simulink model "cddDcmApp"  (R2022b)
settings: Solver=FixedStepDiscrete  StartTime=0.0  StopTime=10.0  FixedStep=0.02  RelTol=1e-3
undefined symbols (not defined in the file or in its folder): CCPFaultType, DcChlType, HFAChlType, IcuChannelType, MtrRoutStaEnum, P_SignalPartType, P_Std_ReturnType, PowerChlType, SrsAdChlType, VGAChlType
(part 8 of 13)

== system cddDcmApp/DataServices_DID_D928_DID_D928_3_ADC_value_Upper_Endstop_at_100/DataServices_DID_D928_DID_D928_3_ADC_value_Upper_Endstop_at_100_ReadData_sys ==
  function : TriggerPort  TriggerType=function-call  IsSimulinkFunction=on  FunctionName=DataServices_DID_D928_DID_D928_3_ADC_value_Upper_Endstop_at_100_ReadData  VariantControl=(inherit)
  Ground_1 : Ground
  Ground_2 : Ground
  Terminator_1 : Terminator
  OpStatus : ArgIn  OutDataTypeStr=Enum: Dcm_OpStatusType  ArgumentName=OpStatus
  Data : ArgOut  OutDataTypeStr=uint8  ArgumentName=Data
  ERR : ArgOut  Port=2  OutDataTypeStr=Std_ReturnType  ArgumentName=ERR
  wires:
  OpStatus/1 -> Terminator_1/1
  Ground_1/1 -> Data/1
  Ground_2/1 -> ERR/1

== system cddDcmApp/DataServices_DID_D928_DID_D928_3_ADC_value_Upper_Endstop_at_100/DataServices_DID_D928_DID_D928_3_ADC_value_Upper_Endstop_at_100_WriteData_sys ==
  function : TriggerPort  TriggerType=function-call  IsSimulinkFunction=on  FunctionName=DataServices_DID_D928_DID_D928_3_ADC_value_Upper_Endstop_at_100_WriteData  VariantControl=(inherit)
  Ground_1 : Ground
  Ground_2 : Ground
  Terminator_1 : Terminator
  Terminator_2 : Terminator
  Data : ArgIn  OutDataTypeStr=uint8  PortDimensions=2  ArgumentName=Data
  OpStatus : ArgIn  Port=2  OutDataTypeStr=Enum: Dcm_OpStatusType  ArgumentName=OpStatus
  ErrorCode : ArgOut  OutDataTypeStr=Enum: Dcm_NegativeResponseCodeType  ArgumentName=ErrorCode
  ERR : ArgOut  Port=2  OutDataTypeStr=Std_ReturnType  ArgumentName=ERR
  wires:
  Data/1 -> Terminator_1/1
  OpStatus/1 -> Terminator_2/1
  Ground_1/1 -> ErrorCode/1
  Ground_2/1 -> ERR/1

== system cddDcmApp/DataServices_DID_D929_ComplexStructure_2/DataServices_DID_D929_ComplexStructure_2_ReadData_sys ==
  function : TriggerPort  TriggerType=function-call  IsSimulinkFunction=on  FunctionName=DataServices_DID_D929_ComplexStructure_2_ReadData  VariantControl=(inherit)
  Ground_1 : Ground
  Ground_2 : Ground
  Terminator_1 : Terminator
  OpStatus : ArgIn  OutDataTypeStr=Enum: Dcm_OpStatusType  ArgumentName=OpStatus
  Data : ArgOut  OutDataTypeStr=uint8  ArgumentName=Data
  ERR : ArgOut  Port=2  OutDataTypeStr=Std_ReturnType  ArgumentName=ERR
  wires:
  OpStatus/1 -> Terminator_1/1
  Ground_1/1 -> Data/1
  Ground_2/1 -> ERR/1

== system cddDcmApp/DataServices_DID_D929_DID_D929_1_Requested_Position/DataServices_DID_D929_DID_D929_1_Requested_Position_ReadData_sys ==
  function : TriggerPort  TriggerType=function-call  IsSimulinkFunction=on  FunctionName=DataServices_DID_D929_DID_D929_1_Requested_Position_ReadData  VariantControl=(inherit)
  Ground_1 : Ground
  Ground_2 : Ground
  Terminator_1 : Terminator
  OpStatus : ArgIn  OutDataTypeStr=Enum: Dcm_OpStatusType  ArgumentName=OpStatus
  Data : ArgOut  OutDataTypeStr=uint8  ArgumentName=Data
  ERR : ArgOut  Port=2  OutDataTypeStr=Std_ReturnType  ArgumentName=ERR
  wires:
  OpStatus/1 -> Terminator_1/1
  Ground_1/1 -> Data/1
  Ground_2/1 -> ERR/1

== system cddDcmApp/DataServices_DID_D929_DID_D929_2_Actual_Position/DataServices_DID_D929_DID_D929_2_Actual_Position_ReadData_sys ==
  function : TriggerPort  TriggerType=function-call  IsSimulinkFunction=on  FunctionName=DataServices_DID_D929_DID_D929_2_Actual_Position_ReadData  VariantControl=(inherit)
  Ground_1 : Ground
  Ground_2 : Ground
  Terminator_1 : Terminator
  OpStatus : ArgIn  OutDataTypeStr=Enum: Dcm_OpStatusType  ArgumentName=OpStatus
  Data : ArgOut  OutDataTypeStr=uint8  ArgumentName=Data
  ERR : ArgOut  Port=2  OutDataTypeStr=Std_ReturnType  ArgumentName=ERR
  wires:
  OpStatus/1 -> Terminator_1/1
  Ground_1/1 -> Data/1
  Ground_2/1 -> ERR/1

== system cddDcmApp/DataServices_DID_D92A_DID_D92A_1_No_Of_Movements/DataServices_DID_D92A_DID_D92A_1_No_Of_Movements_ReadData_sys ==
  function : TriggerPort  TriggerType=function-call  IsSimulinkFunction=on  FunctionName=DataServices_DID_D92A_DID_D92A_1_No_Of_Movements_ReadData  VariantControl=(inherit)
  Ground_1 : Ground
  Ground_2 : Ground
  Terminator_1 : Terminator
  OpStatus : ArgIn  OutDataTypeStr=Enum: Dcm_OpStatusType  ArgumentName=OpStatus
  Data : ArgOut  OutDataTypeStr=uint8  ArgumentName=Data
  ERR : ArgOut  Port=2  OutDataTypeStr=Std_ReturnType  ArgumentName=ERR
  wires:
  OpStatus/1 -> Terminator_1/1
  Ground_1/1 -> Data/1
  Ground_2/1 -> ERR/1

== system cddDcmApp/DataServices_DID_D92A_DID_D92A_1_No_Of_Movements/DataServices_DID_D92A_DID_D92A_1_No_Of_Movements_WriteData_sys ==
  function : TriggerPort  TriggerType=function-call  IsSimulinkFunction=on  FunctionName=DataServices_DID_D92A_DID_D92A_1_No_Of_Movements_WriteData  VariantControl=(inherit)
  Ground_1 : Ground
  Ground_2 : Ground
  Terminator_1 : Terminator
  Terminator_2 : Terminator
  Data : ArgIn  OutDataTypeStr=uint8  PortDimensions=4  ArgumentName=Data
  OpStatus : ArgIn  Port=2  OutDataTypeStr=Enum: Dcm_OpStatusType  ArgumentName=OpStatus
  ErrorCode : ArgOut  OutDataTypeStr=Enum: Dcm_NegativeResponseCodeType  ArgumentName=ErrorCode
  ERR : ArgOut  Port=2  OutDataTypeStr=Std_ReturnType  ArgumentName=ERR
  wires:
  Data/1 -> Terminator_1/1
  OpStatus/1 -> Terminator_2/1
  Ground_1/1 -> ErrorCode/1
  Ground_2/1 -> ERR/1

== system cddDcmApp/DataServices_DID_D92A_DID_D92A_2_ADC_value_Lower_Endstop_at_0/DataServices_DID_D92A_DID_D92A_2_ADC_value_Lower_Endstop_at_0_ReadData_sys ==
  function : TriggerPort  TriggerType=function-call  IsSimulinkFunction=on  FunctionName=DataServices_DID_D92A_DID_D92A_2_ADC_value_Lower_Endstop_at_0_ReadData  VariantControl=(inherit)
  Ground_1 : Ground
  Ground_2 : Ground
  Terminator_1 : Terminator
  OpStatus : ArgIn  OutDataTypeStr=Enum: Dcm_OpStatusType  ArgumentName=OpStatus
  Data : ArgOut  OutDataTypeStr=uint8  ArgumentName=Data
  ERR : ArgOut  Port=2  OutDataTypeStr=Std_ReturnType  ArgumentName=ERR
  wires:
  OpStatus/1 -> Terminator_1/1
  Ground_1/1 -> Data/1
  Ground_2/1 -> ERR/1

== system cddDcmApp/DataServices_DID_D92A_DID_D92A_2_ADC_value_Lower_Endstop_at_0/DataServices_DID_D92A_DID_D92A_2_ADC_value_Lower_Endstop_at_0_WriteData_sys ==
  function : TriggerPort  TriggerType=function-call  IsSimulinkFunction=on  FunctionName=DataServices_DID_D92A_DID_D92A_2_ADC_value_Lower_Endstop_at_0_WriteData  VariantControl=(inherit)
  Ground_1 : Ground
  Ground_2 : Ground
  Terminator_1 : Terminator
  Terminator_2 : Terminator
  Data : ArgIn  OutDataTypeStr=uint8  PortDimensions=2  ArgumentName=Data
  OpStatus : ArgIn  Port=2  OutDataTypeStr=Enum: Dcm_OpStatusType  ArgumentName=OpStatus
  ErrorCode : ArgOut  OutDataTypeStr=Enum: Dcm_NegativeResponseCodeType  ArgumentName=ErrorCode
  ERR : ArgOut  Port=2  OutDataTypeStr=Std_ReturnType  ArgumentName=ERR
  wires:
  Data/1 -> Terminator_1/1
  OpStatus/1 -> Terminator_2/1
  Ground_1/1 -> ErrorCode/1
  Ground_2/1 -> ERR/1

== system cddDcmApp/DataServices_DID_D92A_DID_D92A_3_ADC_value_Upper_Endstop_at_100/DataServices_DID_D92A_DID_D92A_3_ADC_value_Upper_Endstop_at_100_ReadData_sys ==
  function : TriggerPort  TriggerType=function-call  IsSimulinkFunction=on  FunctionName=DataServices_DID_D92A_DID_D92A_3_ADC_value_Upper_Endstop_at_100_ReadData  VariantControl=(inherit)
  Ground_1 : Ground
  Ground_2 : Ground
  Terminator_1 : Terminator
  OpStatus : ArgIn  OutDataTypeStr=Enum: Dcm_OpStatusType  ArgumentName=OpStatus
  Data : ArgOut  OutDataTypeStr=uint8  ArgumentName=Data
  ERR : ArgOut  Port=2  OutDataTypeStr=Std_ReturnType  ArgumentName=ERR
  wires:
  OpStatus/1 -> Terminator_1/1
  Ground_1/1 -> Data/1
  Ground_2/1 -> ERR/1

== system cddDcmApp/DataServices_DID_D92A_DID_D92A_3_ADC_value_Upper_Endstop_at_100/DataServices_DID_D92A_DID_D92A_3_ADC_value_Upper_Endstop_at_100_WriteData_sys ==
  function : TriggerPort  TriggerType=function-call  IsSimulinkFunction=on  FunctionName=DataServices_DID_D92A_DID_D92A_3_ADC_value_Upper_Endstop_at_100_WriteData  VariantControl=(inherit)
  Ground_1 : Ground
  Ground_2 : Ground
  Terminator_1 : Terminator
  Terminator_2 : Terminator
  Data : ArgIn  OutDataTypeStr=uint8  PortDimensions=2  ArgumentName=Data
  OpStatus : ArgIn  Port=2  OutDataTypeStr=Enum: Dcm_OpStatusType  ArgumentName=OpStatus
  ErrorCode : ArgOut  OutDataTypeStr=Enum: Dcm_NegativeResponseCodeType  ArgumentName=ErrorCode
  ERR : ArgOut  Port=2  OutDataTypeStr=Std_ReturnType  ArgumentName=ERR
  wires:
  Data/1 -> Terminator_1/1
  OpStatus/1 -> Terminator_2/1
  Ground_1/1 -> ErrorCode/1
  Ground_2/1 -> ERR/1

== system cddDcmApp/DataServices_DID_D92B_ComplexStructure_2/DataServices_DID_D92B_ComplexStructure_2_ReadData_sys ==
  function : TriggerPort  TriggerType=function-call  IsSimulinkFunction=on  FunctionName=DataServices_DID_D92B_ComplexStructure_2_ReadData  VariantControl=(inherit)
  Ground_1 : Ground
  Ground_2 : Ground
  Terminator_1 : Terminator
  OpStatus : ArgIn  OutDataTypeStr=Enum: Dcm_OpStatusType  ArgumentName=OpStatus
  Data : ArgOut  OutDataTypeStr=uint8  ArgumentName=Data
  ERR : ArgOut  Port=2  OutDataTypeStr=Std_ReturnType  ArgumentName=ERR
  wires:
  OpStatus/1 -> Terminator_1/1
  Ground_1/1 -> Data/1
  Ground_2/1 -> ERR/1

== system cddDcmApp/DataServices_DID_D92B_DID_D92B_1_Requested_Position/DataServices_DID_D92B_DID_D92B_1_Requested_Position_ReadData_sys ==
  function : TriggerPort  TriggerType=function-call  IsSimulinkFunction=on  FunctionName=DataServices_DID_D92B_DID_D92B_1_Requested_Position_ReadData  VariantControl=(inherit)
  Ground_1 : Ground
  Ground_2 : Ground
  Terminator_1 : Terminator
  OpStatus : ArgIn  OutDataTypeStr=Enum: Dcm_OpStatusType  ArgumentName=OpStatus
  Data : ArgOut  OutDataTypeStr=uint8  ArgumentName=Data
  ERR : ArgOut  Port=2  OutDataTypeStr=Std_ReturnType  ArgumentName=ERR
  wires:
  OpStatus/1 -> Terminator_1/1
  Ground_1/1 -> Data/1
  Ground_2/1 -> ERR/1

== system cddDcmApp/DataServices_DID_D92B_DID_D92B_2_Actual_Position/DataServices_DID_D92B_DID_D92B_2_Actual_Position_ReadData_sys ==
  function : TriggerPort  TriggerType=function-call  IsSimulinkFunction=on  FunctionName=DataServices_DID_D92B_DID_D92B_2_Actual_Position_ReadData  VariantControl=(inherit)
  Ground_1 : Ground
  Ground_2 : Ground
  Terminator_1 : Terminator
  OpStatus : ArgIn  OutDataTypeStr=Enum: Dcm_OpStatusType  ArgumentName=OpStatus
  Data : ArgOut  OutDataTypeStr=uint8  ArgumentName=Data
  ERR : ArgOut  Port=2  OutDataTypeStr=Std_ReturnType  ArgumentName=ERR
  wires:
  OpStatus/1 -> Terminator_1/1
  Ground_1/1 -> Data/1
  Ground_2/1 -> ERR/1

== system cddDcmApp/DataServices_DID_D92C_DID_D92C_1_No_Of_Movements/DataServices_DID_D92C_DID_D92C_1_No_Of_Movements_ReadData_sys ==
  function : TriggerPort  TriggerType=function-call  IsSimulinkFunction=on  FunctionName=DataServices_DID_D92C_DID_D92C_1_No_Of_Movements_ReadData  VariantControl=(inherit)
  Ground_1 : Ground
  Ground_2 : Ground
  Terminator_1 : Terminator
  OpStatus : ArgIn  OutDataTypeStr=Enum: Dcm_OpStatusType  ArgumentName=OpStatus
  Data : ArgOut  OutDataTypeStr=uint8  ArgumentName=Data
  ERR : ArgOut  Port=2  OutDataTypeStr=Std_ReturnType  ArgumentName=ERR
  wires:
  OpStatus/1 -> Terminator_1/1
  Ground_1/1 -> Data/1
  Ground_2/1 -> ERR/1

== system cddDcmApp/DataServices_DID_D92C_DID_D92C_1_No_Of_Movements/DataServices_DID_D92C_DID_D92C_1_No_Of_Movements_WriteData_sys ==
  function : TriggerPort  TriggerType=function-call  IsSimulinkFunction=on  FunctionName=DataServices_DID_D92C_DID_D92C_1_No_Of_Movements_WriteData  VariantControl=(inherit)
  Ground_1 : Ground
  Ground_2 : Ground
  Terminator_1 : Terminator
  Terminator_2 : Terminator
  Data : ArgIn  OutDataTypeStr=uint8  PortDimensions=4  ArgumentName=Data
  OpStatus : ArgIn  Port=2  OutDataTypeStr=Enum: Dcm_OpStatusType  ArgumentName=OpStatus
  ErrorCode : ArgOut  OutDataTypeStr=Enum: Dcm_NegativeResponseCodeType  ArgumentName=ErrorCode
  ERR : ArgOut  Port=2  OutDataTypeStr=Std_ReturnType  ArgumentName=ERR
  wires:
  Data/1 -> Terminator_1/1
  OpStatus/1 -> Terminator_2/1
  Ground_1/1 -> ErrorCode/1
  Ground_2/1 -> ERR/1

== system cddDcmApp/DataServices_DID_D92C_DID_D92C_2_ADC_value_Lower_Endstop_at_0/DataServices_DID_D92C_DID_D92C_2_ADC_value_Lower_Endstop_at_0_ReadData_sys ==
  function : TriggerPort  TriggerType=function-call  IsSimulinkFunction=on  FunctionName=DataServices_DID_D92C_DID_D92C_2_ADC_value_Lower_Endstop_at_0_ReadData  VariantControl=(inherit)
  Ground_1 : Ground
  Ground_2 : Ground
  Terminator_1 : Terminator
  OpStatus : ArgIn  OutDataTypeStr=Enum: Dcm_OpStatusType  ArgumentName=OpStatus
  Data : ArgOut  OutDataTypeStr=uint8  ArgumentName=Data
  ERR : ArgOut  Port=2  OutDataTypeStr=Std_ReturnType  ArgumentName=ERR
  wires:
  OpStatus/1 -> Terminator_1/1
  Ground_1/1 -> Data/1
  Ground_2/1 -> ERR/1

== system cddDcmApp/DataServices_DID_D92C_DID_D92C_2_ADC_value_Lower_Endstop_at_0/DataServices_DID_D92C_DID_D92C_2_ADC_value_Lower_Endstop_at_0_WriteData_sys ==
  function : TriggerPort  TriggerType=function-call  IsSimulinkFunction=on  FunctionName=DataServices_DID_D92C_DID_D92C_2_ADC_value_Lower_Endstop_at_0_WriteData  VariantControl=(inherit)
  Ground_1 : Ground
  Ground_2 : Ground
  Terminator_1 : Terminator
  Terminator_2 : Terminator
  Data : ArgIn  OutDataTypeStr=uint8  PortDimensions=2  ArgumentName=Data
  OpStatus : ArgIn  Port=2  OutDataTypeStr=Enum: Dcm_OpStatusType  ArgumentName=OpStatus
  ErrorCode : ArgOut  OutDataTypeStr=Enum: Dcm_NegativeResponseCodeType  ArgumentName=ErrorCode
  ERR : ArgOut  Port=2  OutDataTypeStr=Std_ReturnType  ArgumentName=ERR
  wires:
  Data/1 -> Terminator_1/1
  OpStatus/1 -> Terminator_2/1
  Ground_1/1 -> ErrorCode/1
  Ground_2/1 -> ERR/1

== system cddDcmApp/DataServices_DID_D92C_DID_D92C_3_ADC_value_Upper_Endstop_at_100/DataServices_DID_D92C_DID_D92C_3_ADC_value_Upper_Endstop_at_100_ReadData_sys ==
  function : TriggerPort  TriggerType=function-call  IsSimulinkFunction=on  FunctionName=DataServices_DID_D92C_DID_D92C_3_ADC_value_Upper_Endstop_at_100_ReadData  VariantControl=(inherit)
  Ground_1 : Ground
  Ground_2 : Ground
  Terminator_1 : Terminator
  OpStatus : ArgIn  OutDataTypeStr=Enum: Dcm_OpStatusType  ArgumentName=OpStatus
  Data : ArgOut  OutDataTypeStr=uint8  ArgumentName=Data
  ERR : ArgOut  Port=2  OutDataTypeStr=Std_ReturnType  ArgumentName=ERR
  wires:
  OpStatus/1 -> Terminator_1/1
  Ground_1/1 -> Data/1
  Ground_2/1 -> ERR/1

== system cddDcmApp/DataServices_DID_D92C_DID_D92C_3_ADC_value_Upper_Endstop_at_100/DataServices_DID_D92C_DID_D92C_3_ADC_value_Upper_Endstop_at_100_WriteData_sys ==
  function : TriggerPort  TriggerType=function-call  IsSimulinkFunction=on  FunctionName=DataServices_DID_D92C_DID_D92C_3_ADC_value_Upper_Endstop_at_100_WriteData  VariantControl=(inherit)
  Ground_1 : Ground
  Ground_2 : Ground
  Terminator_1 : Terminator
  Terminator_2 : Terminator
  Data : ArgIn  OutDataTypeStr=uint8  PortDimensions=2  ArgumentName=Data
  OpStatus : ArgIn  Port=2  OutDataTypeStr=Enum: Dcm_OpStatusType  ArgumentName=OpStatus
  ErrorCode : ArgOut  OutDataTypeStr=Enum: Dcm_NegativeResponseCodeType  ArgumentName=ErrorCode
  ERR : ArgOut  Port=2  OutDataTypeStr=Std_ReturnType  ArgumentName=ERR
  wires:
  Data/1 -> Terminator_1/1
  OpStatus/1 -> Terminator_2/1
  Ground_1/1 -> ErrorCode/1
  Ground_2/1 -> ERR/1

== system cddDcmApp/DataServices_DID_D92D_ComplexStructure_2/DataServices_DID_D92D_ComplexStructure_2_ReadData_sys ==
  function : TriggerPort  TriggerType=function-call  IsSimulinkFunction=on  FunctionName=DataServices_DID_D92D_ComplexStructure_2_ReadData  VariantControl=(inherit)
  Ground_1 : Ground
  Ground_2 : Ground
  Terminator_1 : Terminator
  OpStatus : ArgIn  OutDataTypeStr=Enum: Dcm_OpStatusType  ArgumentName=OpStatus
  Data : ArgOut  OutDataTypeStr=uint8  ArgumentName=Data
  ERR : ArgOut  Port=2  OutDataTypeStr=Std_ReturnType  ArgumentName=ERR
  wires:
  OpStatus/1 -> Terminator_1/1
  Ground_1/1 -> Data/1
  Ground_2/1 -> ERR/1

== system cddDcmApp/DataServices_DID_D92D_DID_D92D_1_Requested_Position/DataServices_DID_D92D_DID_D92D_1_Requested_Position_ReadData_sys ==
  function : TriggerPort  TriggerType=function-call  IsSimulinkFunction=on  FunctionName=DataServices_DID_D92D_DID_D92D_1_Requested_Position_ReadData  VariantControl=(inherit)
  Ground_1 : Ground
  Ground_2 : Ground
  Terminator_1 : Terminator
  OpStatus : ArgIn  OutDataTypeStr=Enum: Dcm_OpStatusType  ArgumentName=OpStatus
  Data : ArgOut  OutDataTypeStr=uint8  ArgumentName=Data
  ERR : ArgOut  Port=2  OutDataTypeStr=Std_ReturnType  ArgumentName=ERR
  wires:
  OpStatus/1 -> Terminator_1/1
  Ground_1/1 -> Data/1
  Ground_2/1 -> ERR/1

== system cddDcmApp/DataServices_DID_D92D_DID_D92D_2_Actual_Position/DataServices_DID_D92D_DID_D92D_2_Actual_Position_ReadData_sys ==
  function : TriggerPort  TriggerType=function-call  IsSimulinkFunction=on  FunctionName=DataServices_DID_D92D_DID_D92D_2_Actual_Position_ReadData  VariantControl=(inherit)
  Ground_1 : Ground
  Ground_2 : Ground
  Terminator_1 : Terminator
  OpStatus : ArgIn  OutDataTypeStr=Enum: Dcm_OpStatusType  ArgumentName=OpStatus
  Data : ArgOut  OutDataTypeStr=uint8  ArgumentName=Data
  ERR : ArgOut  Port=2  OutDataTypeStr=Std_ReturnType  ArgumentName=ERR
  wires:
  OpStatus/1 -> Terminator_1/1
  Ground_1/1 -> Data/1
  Ground_2/1 -> ERR/1

== system cddDcmApp/DataServices_DID_D92E_DID_D92E_1_No_Of_Movements/DataServices_DID_D92E_DID_D92E_1_No_Of_Movements_ReadData_sys ==
  function : TriggerPort  TriggerType=function-call  IsSimulinkFunction=on  FunctionName=DataServices_DID_D92E_DID_D92E_1_No_Of_Movements_ReadData  VariantControl=(inherit)
  Ground_1 : Ground
  Ground_2 : Ground
  Terminator_1 : Terminator
  OpStatus : ArgIn  OutDataTypeStr=Enum: Dcm_OpStatusType  ArgumentName=OpStatus
  Data : ArgOut  OutDataTypeStr=uint8  ArgumentName=Data
  ERR : ArgOut  Port=2  OutDataTypeStr=Std_ReturnType  ArgumentName=ERR
  wires:
  OpStatus/1 -> Terminator_1/1
  Ground_1/1 -> Data/1
  Ground_2/1 -> ERR/1

== system cddDcmApp/DataServices_DID_D92E_DID_D92E_1_No_Of_Movements/DataServices_DID_D92E_DID_D92E_1_No_Of_Movements_WriteData_sys ==
  function : TriggerPort  TriggerType=function-call  IsSimulinkFunction=on  FunctionName=DataServices_DID_D92E_DID_D92E_1_No_Of_Movements_WriteData  VariantControl=(inherit)
  Ground_1 : Ground
  Ground_2 : Ground
  Terminator_1 : Terminator
  Terminator_2 : Terminator
  Data : ArgIn  OutDataTypeStr=uint8  PortDimensions=4  ArgumentName=Data
  OpStatus : ArgIn  Port=2  OutDataTypeStr=Enum: Dcm_OpStatusType  ArgumentName=OpStatus
  ErrorCode : ArgOut  OutDataTypeStr=Enum: Dcm_NegativeResponseCodeType  ArgumentName=ErrorCode
  ERR : ArgOut  Port=2  OutDataTypeStr=Std_ReturnType  ArgumentName=ERR
  wires:
  Data/1 -> Terminator_1/1
  OpStatus/1 -> Terminator_2/1
  Ground_1/1 -> ErrorCode/1
  Ground_2/1 -> ERR/1

== system cddDcmApp/DataServices_DID_D92E_DID_D92E_2_ADC_value_Lower_Endstop_at_0/DataServices_DID_D92E_DID_D92E_2_ADC_value_Lower_Endstop_at_0_ReadData_sys ==
  function : TriggerPort  TriggerType=function-call  IsSimulinkFunction=on  FunctionName=DataServices_DID_D92E_DID_D92E_2_ADC_value_Lower_Endstop_at_0_ReadData  VariantControl=(inherit)
  Ground_1 : Ground
  Ground_2 : Ground
  Terminator_1 : Terminator
  OpStatus : ArgIn  OutDataTypeStr=Enum: Dcm_OpStatusType  ArgumentName=OpStatus
  Data : ArgOut  OutDataTypeStr=uint8  ArgumentName=Data
  ERR : ArgOut  Port=2  OutDataTypeStr=Std_ReturnType  ArgumentName=ERR
  wires:
  OpStatus/1 -> Terminator_1/1
  Ground_1/1 -> Data/1
  Ground_2/1 -> ERR/1

== system cddDcmApp/DataServices_DID_D92E_DID_D92E_2_ADC_value_Lower_Endstop_at_0/DataServices_DID_D92E_DID_D92E_2_ADC_value_Lower_Endstop_at_0_WriteData_sys ==
  function : TriggerPort  TriggerType=function-call  IsSimulinkFunction=on  FunctionName=DataServices_DID_D92E_DID_D92E_2_ADC_value_Lower_Endstop_at_0_WriteData  VariantControl=(inherit)
  Ground_1 : Ground
  Ground_2 : Ground
  Terminator_1 : Terminator
  Terminator_2 : Terminator
  Data : ArgIn  OutDataTypeStr=uint8  PortDimensions=2  ArgumentName=Data
  OpStatus : ArgIn  Port=2  OutDataTypeStr=Enum: Dcm_OpStatusType  ArgumentName=OpStatus
  ErrorCode : ArgOut  OutDataTypeStr=Enum: Dcm_NegativeResponseCodeType  ArgumentName=ErrorCode
  ERR : ArgOut  Port=2  OutDataTypeStr=Std_ReturnType  ArgumentName=ERR
  wires:
  Data/1 -> Terminator_1/1
  OpStatus/1 -> Terminator_2/1
  Ground_1/1 -> ErrorCode/1
  Ground_2/1 -> ERR/1

== system cddDcmApp/DataServices_DID_D92E_DID_D92E_3_ADC_value_Upper_Endstop_at_100/DataServices_DID_D92E_DID_D92E_3_ADC_value_Upper_Endstop_at_100_ReadData_sys ==
  function : TriggerPort  TriggerType=function-call  IsSimulinkFunction=on  FunctionName=DataServices_DID_D92E_DID_D92E_3_ADC_value_Upper_Endstop_at_100_ReadData  VariantControl=(inherit)
  Ground_1 : Ground
  Ground_2 : Ground
  Terminator_1 : Terminator
  OpStatus : ArgIn  OutDataTypeStr=Enum: Dcm_OpStatusType  ArgumentName=OpStatus
  Data : ArgOut  OutDataTypeStr=uint8  ArgumentName=Data
  ERR : ArgOut  Port=2  OutDataTypeStr=Std_ReturnType  ArgumentName=ERR
  wires:
  OpStatus/1 -> Terminator_1/1
  Ground_1/1 -> Data/1
  Ground_2/1 -> ERR/1

== system cddDcmApp/DataServices_DID_D92E_DID_D92E_3_ADC_value_Upper_Endstop_at_100/DataServices_DID_D92E_DID_D92E_3_ADC_value_Upper_Endstop_at_100_WriteData_sys ==
  function : TriggerPort  TriggerType=function-call  IsSimulinkFunction=on  FunctionName=DataServices_DID_D92E_DID_D92E_3_ADC_value_Upper_Endstop_at_100_WriteData  VariantControl=(inherit)
  Ground_1 : Ground
  Ground_2 : Ground
  Terminator_1 : Terminator
  Terminator_2 : Terminator
  Data : ArgIn  OutDataTypeStr=uint8  PortDimensions=2  ArgumentName=Data
  OpStatus : ArgIn  Port=2  OutDataTypeStr=Enum: Dcm_OpStatusType  ArgumentName=OpStatus
  ErrorCode : ArgOut  OutDataTypeStr=Enum: Dcm_NegativeResponseCodeType  ArgumentName=ErrorCode
  ERR : ArgOut  Port=2  OutDataTypeStr=Std_ReturnType  ArgumentName=ERR
  wires:
  Data/1 -> Terminator_1/1
  OpStatus/1 -> Terminator_2/1
  Ground_1/1 -> ErrorCode/1
  Ground_2/1 -> ERR/1

== system cddDcmApp/DataServices_DID_D92F_ComplexStructure_2/DataServices_DID_D92F_ComplexStructure_2_ReadData_sys ==
  function : TriggerPort  TriggerType=function-call  IsSimulinkFunction=on  FunctionName=DataServices_DID_D92F_ComplexStructure_2_ReadData  VariantControl=(inherit)
  Ground_1 : Ground
  Ground_2 : Ground
  Terminator_1 : Terminator
  OpStatus : ArgIn  OutDataTypeStr=Enum: Dcm_OpStatusType  ArgumentName=OpStatus
  Data : ArgOut  OutDataTypeStr=uint8  ArgumentName=Data
  ERR : ArgOut  Port=2  OutDataTypeStr=Std_ReturnType  ArgumentName=ERR
  wires:
  OpStatus/1 -> Terminator_1/1
  Ground_1/1 -> Data/1
  Ground_2/1 -> ERR/1

== system cddDcmApp/DataServices_DID_D92F_DID_D92F_1_Requested_Position/DataServices_DID_D92F_DID_D92F_1_Requested_Position_ReadData_sys ==
  function : TriggerPort  TriggerType=function-call  IsSimulinkFunction=on  FunctionName=DataServices_DID_D92F_DID_D92F_1_Requested_Position_ReadData  VariantControl=(inherit)
  Ground_1 : Ground
  Ground_2 : Ground
  Terminator_1 : Terminator
  OpStatus : ArgIn  OutDataTypeStr=Enum: Dcm_OpStatusType  ArgumentName=OpStatus
  Data : ArgOut  OutDataTypeStr=uint8  ArgumentName=Data
  ERR : ArgOut  Port=2  OutDataTypeStr=Std_ReturnType  ArgumentName=ERR
  wires:
  OpStatus/1 -> Terminator_1/1
  Ground_1/1 -> Data/1
  Ground_2/1 -> ERR/1

== system cddDcmApp/DataServices_DID_D92F_DID_D92F_2_Actual_Position/DataServices_DID_D92F_DID_D92F_2_Actual_Position_ReadData_sys ==
  function : TriggerPort  TriggerType=function-call  IsSimulinkFunction=on  FunctionName=DataServices_DID_D92F_DID_D92F_2_Actual_Position_ReadData  VariantControl=(inherit)
  Ground_1 : Ground
  Ground_2 : Ground
  Terminator_1 : Terminator
  OpStatus : ArgIn  OutDataTypeStr=Enum: Dcm_OpStatusType  ArgumentName=OpStatus
  Data : ArgOut  OutDataTypeStr=uint8  ArgumentName=Data
  ERR : ArgOut  Port=2  OutDataTypeStr=Std_ReturnType  ArgumentName=ERR
  wires:
  OpStatus/1 -> Terminator_1/1
  Ground_1/1 -> Data/1
  Ground_2/1 -> ERR/1

== system cddDcmApp/DataServices_DID_D930_DID_D930_1_No_Of_Movements/DataServices_DID_D930_DID_D930_1_No_Of_Movements_ReadData_sys ==
  function : TriggerPort  TriggerType=function-call  IsSimulinkFunction=on  FunctionName=DataServices_DID_D930_DID_D930_1_No_Of_Movements_ReadData  VariantControl=(inherit)
  Ground_1 : Ground
  Ground_2 : Ground
  Terminator_1 : Terminator
  OpStatus : ArgIn  OutDataTypeStr=Enum: Dcm_OpStatusType  ArgumentName=OpStatus
  Data : ArgOut  OutDataTypeStr=uint8  ArgumentName=Data
  ERR : ArgOut  Port=2  OutDataTypeStr=Std_ReturnType  ArgumentName=ERR
  wires:
  OpStatus/1 -> Terminator_1/1
  Ground_1/1 -> Data/1
  Ground_2/1 -> ERR/1

== system cddDcmApp/DataServices_DID_D930_DID_D930_1_No_Of_Movements/DataServices_DID_D930_DID_D930_1_No_Of_Movements_WriteData_sys ==
  function : TriggerPort  TriggerType=function-call  IsSimulinkFunction=on  FunctionName=DataServices_DID_D930_DID_D930_1_No_Of_Movements_WriteData  VariantControl=(inherit)
  Ground_1 : Ground
  Ground_2 : Ground
  Terminator_1 : Terminator
  Terminator_2 : Terminator
  Data : ArgIn  OutDataTypeStr=uint8  PortDimensions=4  ArgumentName=Data
  OpStatus : ArgIn  Port=2  OutDataTypeStr=Enum: Dcm_OpStatusType  ArgumentName=OpStatus
  ErrorCode : ArgOut  OutDataTypeStr=Enum: Dcm_NegativeResponseCodeType  ArgumentName=ErrorCode
  ERR : ArgOut  Port=2  OutDataTypeStr=Std_ReturnType  ArgumentName=ERR
  wires:
  Data/1 -> Terminator_1/1
  OpStatus/1 -> Terminator_2/1
  Ground_1/1 -> ErrorCode/1
  Ground_2/1 -> ERR/1

== system cddDcmApp/DataServices_DID_D930_DID_D930_2_ADC_value_Lower_Endstop_at_0/DataServices_DID_D930_DID_D930_2_ADC_value_Lower_Endstop_at_0_ReadData_sys ==
  function : TriggerPort  TriggerType=function-call  IsSimulinkFunction=on  FunctionName=DataServices_DID_D930_DID_D930_2_ADC_value_Lower_Endstop_at_0_ReadData  VariantControl=(inherit)
  Ground_1 : Ground
  Ground_2 : Ground
  Terminator_1 : Terminator
  OpStatus : ArgIn  OutDataTypeStr=Enum: Dcm_OpStatusType  ArgumentName=OpStatus
  Data : ArgOut  OutDataTypeStr=uint8  ArgumentName=Data
  ERR : ArgOut  Port=2  OutDataTypeStr=Std_ReturnType  ArgumentName=ERR
  wires:
  OpStatus/1 -> Terminator_1/1
  Ground_1/1 -> Data/1
  Ground_2/1 -> ERR/1

== system cddDcmApp/DataServices_DID_D930_DID_D930_2_ADC_value_Lower_Endstop_at_0/DataServices_DID_D930_DID_D930_2_ADC_value_Lower_Endstop_at_0_WriteData_sys ==
  function : TriggerPort  TriggerType=function-call  IsSimulinkFunction=on  FunctionName=DataServices_DID_D930_DID_D930_2_ADC_value_Lower_Endstop_at_0_WriteData  VariantControl=(inherit)
  Ground_1 : Ground
  Ground_2 : Ground
  Terminator_1 : Terminator
  Terminator_2 : Terminator
  Data : ArgIn  OutDataTypeStr=uint8  PortDimensions=2  ArgumentName=Data
  OpStatus : ArgIn  Port=2  OutDataTypeStr=Enum: Dcm_OpStatusType  ArgumentName=OpStatus
  ErrorCode : ArgOut  OutDataTypeStr=Enum: Dcm_NegativeResponseCodeType  ArgumentName=ErrorCode
  ERR : ArgOut  Port=2  OutDataTypeStr=Std_ReturnType  ArgumentName=ERR
  wires:
  Data/1 -> Terminator_1/1
  OpStatus/1 -> Terminator_2/1
  Ground_1/1 -> ErrorCode/1
  Ground_2/1 -> ERR/1

== system cddDcmApp/DataServices_DID_D930_DID_D930_3_ADC_value_Upper_Endstop_at_100/DataServices_DID_D930_DID_D930_3_ADC_value_Upper_Endstop_at_100_ReadData_sys ==
  function : TriggerPort  TriggerType=function-call  IsSimulinkFunction=on  FunctionName=DataServices_DID_D930_DID_D930_3_ADC_value_Upper_Endstop_at_100_ReadData  VariantControl=(inherit)
  Ground_1 : Ground
  Ground_2 : Ground
  Terminator_1 : Terminator
  OpStatus : ArgIn  OutDataTypeStr=Enum: Dcm_OpStatusType  ArgumentName=OpStatus
  Data : ArgOut  OutDataTypeStr=uint8  ArgumentName=Data
  ERR : ArgOut  Port=2  OutDataTypeStr=Std_ReturnType  ArgumentName=ERR
  wires:
  OpStatus/1 -> Terminator_1/1
  Ground_1/1 -> Data/1
  Ground_2/1 -> ERR/1

== system cddDcmApp/DataServices_DID_D930_DID_D930_3_ADC_value_Upper_Endstop_at_100/DataServices_DID_D930_DID_D930_3_ADC_value_Upper_Endstop_at_100_WriteData_sys ==
  function : TriggerPort  TriggerType=function-call  IsSimulinkFunction=on  FunctionName=DataServices_DID_D930_DID_D930_3_ADC_value_Upper_Endstop_at_100_WriteData  VariantControl=(inherit)
  Ground_1 : Ground
  Ground_2 : Ground
  Terminator_1 : Terminator
  Terminator_2 : Terminator
  Data : ArgIn  OutDataTypeStr=uint8  PortDimensions=2  ArgumentName=Data
  OpStatus : ArgIn  Port=2  OutDataTypeStr=Enum: Dcm_OpStatusType  ArgumentName=OpStatus
  ErrorCode : ArgOut  OutDataTypeStr=Enum: Dcm_NegativeResponseCodeType  ArgumentName=ErrorCode
  ERR : ArgOut  Port=2  OutDataTypeStr=Std_ReturnType  ArgumentName=ERR
  wires:
  Data/1 -> Terminator_1/1
  OpStatus/1 -> Terminator_2/1
  Ground_1/1 -> ErrorCode/1
  Ground_2/1 -> ERR/1

== system cddDcmApp/DataServices_DID_D931_ComplexStructure_2/DataServices_DID_D931_ComplexStructure_2_ReadData_sys ==
  function : TriggerPort  TriggerType=function-call  IsSimulinkFunction=on  FunctionName=DataServices_DID_D931_ComplexStructure_2_ReadData  VariantControl=(inherit)
  Ground_1 : Ground
  Ground_2 : Ground
  Terminator_1 : Terminator
  OpStatus : ArgIn  OutDataTypeStr=Enum: Dcm_OpStatusType  ArgumentName=OpStatus
  Data : ArgOut  OutDataTypeStr=uint8  ArgumentName=Data
  ERR : ArgOut  Port=2  OutDataTypeStr=Std_ReturnType  ArgumentName=ERR
  wires:
  OpStatus/1 -> Terminator_1/1
  Ground_1/1 -> Data/1
  Ground_2/1 -> ERR/1

== system cddDcmApp/DataServices_DID_D931_DID_D931_1_Requested_Position/DataServices_DID_D931_DID_D931_1_Requested_Position_ReadData_sys ==
  function : TriggerPort  TriggerType=function-call  IsSimulinkFunction=on  FunctionName=DataServices_DID_D931_DID_D931_1_Requested_Position_ReadData  VariantControl=(inherit)
  Ground_1 : Ground
  Ground_2 : Ground
  Terminator_1 : Terminator
  OpStatus : ArgIn  OutDataTypeStr=Enum: Dcm_OpStatusType  ArgumentName=OpStatus
  Data : ArgOut  OutDataTypeStr=uint8  ArgumentName=Data
  ERR : ArgOut  Port=2  OutDataTypeStr=Std_ReturnType  ArgumentName=ERR
  wires:
  OpStatus/1 -> Terminator_1/1
  Ground_1/1 -> Data/1
  Ground_2/1 -> ERR/1

== system cddDcmApp/DataServices_DID_D931_DID_D931_2_Actual_Position/DataServices_DID_D931_DID_D931_2_Actual_Position_ReadData_sys ==
  function : TriggerPort  TriggerType=function-call  IsSimulinkFunction=on  FunctionName=DataServices_DID_D931_DID_D931_2_Actual_Position_ReadData  VariantControl=(inherit)
  Ground_1 : Ground
  Ground_2 : Ground
  Terminator_1 : Terminator
  OpStatus : ArgIn  OutDataTypeStr=Enum: Dcm_OpStatusType  ArgumentName=OpStatus
  Data : ArgOut  OutDataTypeStr=uint8  ArgumentName=Data
  ERR : ArgOut  Port=2  OutDataTypeStr=Std_ReturnType  ArgumentName=ERR
  wires:
  OpStatus/1 -> Terminator_1/1
  Ground_1/1 -> Data/1
  Ground_2/1 -> ERR/1

== system cddDcmApp/DataServices_DID_D933_DID_D933_1_step_Range/DataServices_DID_D933_DID_D933_1_step_Range_ReadData_sys ==
  function : TriggerPort  TriggerType=function-call  IsSimulinkFunction=on  FunctionName=DataServices_DID_D933_DID_D933_1_step_Range_ReadData  VariantControl=(inherit)
  Ground_1 : Ground
  Ground_2 : Ground
  Terminator_1 : Terminator
  OpStatus : ArgIn  OutDataTypeStr=Enum: Dcm_OpStatusType  ArgumentName=OpStatus
  Data : ArgOut  OutDataTypeStr=uint8  ArgumentName=Data
  ERR : ArgOut  Port=2  OutDataTypeStr=Std_ReturnType  ArgumentName=ERR
  wires:
  OpStatus/1 -> Terminator_1/1
  Ground_1/1 -> Data/1
  Ground_2/1 -> ERR/1

== system cddDcmApp/DataServices_DID_D933_DID_D933_1_step_Range/DataServices_DID_D933_DID_D933_1_step_Range_WriteData_sys ==
  function : TriggerPort  TriggerType=function-call  IsSimulinkFunction=on  FunctionName=DataServices_DID_D933_DID_D933_1_step_Range_WriteData  VariantControl=(inherit)
  Ground_1 : Ground
  Ground_2 : Ground
  Terminator_1 : Terminator
  Terminator_2 : Terminator
  Data : ArgIn  OutDataTypeStr=uint8  PortDimensions=2  ArgumentName=Data
  OpStatus : ArgIn  Port=2  OutDataTypeStr=Enum: Dcm_OpStatusType  ArgumentName=OpStatus
  ErrorCode : ArgOut  OutDataTypeStr=Enum: Dcm_NegativeResponseCodeType  ArgumentName=ErrorCode
  ERR : ArgOut  Port=2  OutDataTypeStr=Std_ReturnType  ArgumentName=ERR
  wires:
  Data/1 -> Terminator_1/1
  OpStatus/1 -> Terminator_2/1
  Ground_1/1 -> ErrorCode/1
  Ground_2/1 -> ERR/1

== system cddDcmApp/DataServices_DID_D933_DID_D933_2_No_Of_Movements/DataServices_DID_D933_DID_D933_2_No_Of_Movements_ReadData_sys ==
  function : TriggerPort  TriggerType=function-call  IsSimulinkFunction=on  FunctionName=DataServices_DID_D933_DID_D933_2_No_Of_Movements_ReadData  VariantControl=(inherit)
  Ground_1 : Ground
  Ground_2 : Ground
  Terminator_1 : Terminator
  OpStatus : ArgIn  OutDataTypeStr=Enum: Dcm_OpStatusType  ArgumentName=OpStatus
  Data : ArgOut  OutDataTypeStr=uint8  ArgumentName=Data
  ERR : ArgOut  Port=2  OutDataTypeStr=Std_ReturnType  ArgumentName=ERR
  wires:
  OpStatus/1 -> Terminator_1/1
  Ground_1/1 -> Data/1
  Ground_2/1 -> ERR/1

== system cddDcmApp/DataServices_DID_D933_DID_D933_2_No_Of_Movements/DataServices_DID_D933_DID_D933_2_No_Of_Movements_WriteData_sys ==
  function : TriggerPort  TriggerType=function-call  IsSimulinkFunction=on  FunctionName=DataServices_DID_D933_DID_D933_2_No_Of_Movements_WriteData  VariantControl=(inherit)
  Ground_1 : Ground
  Ground_2 : Ground
  Terminator_1 : Terminator
  Terminator_2 : Terminator
  Data : ArgIn  OutDataTypeStr=uint8  PortDimensions=4  ArgumentName=Data
  OpStatus : ArgIn  Port=2  OutDataTypeStr=Enum: Dcm_OpStatusType  ArgumentName=OpStatus
  ErrorCode : ArgOut  OutDataTypeStr=Enum: Dcm_NegativeResponseCodeType  ArgumentName=ErrorCode
  ERR : ArgOut  Port=2  OutDataTypeStr=Std_ReturnType  ArgumentName=ERR
  wires:
  Data/1 -> Terminator_1/1
  OpStatus/1 -> Terminator_2/1
  Ground_1/1 -> ErrorCode/1
  Ground_2/1 -> ERR/1

== system cddDcmApp/DataServices_DID_D934_ComplexStructure_2/DataServices_DID_D934_ComplexStructure_2_ReadData_sys ==
  function : TriggerPort  TriggerType=function-call  IsSimulinkFunction=on  FunctionName=DataServices_DID_D934_ComplexStructure_2_ReadData  VariantControl=(inherit)
  Ground_1 : Ground
  Ground_2 : Ground
  Data : ArgOut  OutDataTypeStr=uint8  ArgumentName=Data
  ERR : ArgOut  Port=2  OutDataTypeStr=Std_ReturnType  ArgumentName=ERR
  wires:
  Ground_1/1 -> Data/1
  Ground_2/1 -> ERR/1

== system cddDcmApp/DataServices_DID_D934_DID_D934_1_Requested_Position/DataServices_DID_D934_DID_D934_1_Requested_Position_ReadData_sys ==
  function : TriggerPort  TriggerType=function-call  IsSimulinkFunction=on  FunctionName=DataServices_DID_D934_DID_D934_1_Requested_Position_ReadData  VariantControl=(inherit)
  Ground_1 : Ground
  Ground_2 : Ground
  Data : ArgOut  OutDataTypeStr=uint8  ArgumentName=Data
  ERR : ArgOut  Port=2  OutDataTypeStr=Std_ReturnType  ArgumentName=ERR
  wires:
  Ground_1/1 -> Data/1
  Ground_2/1 -> ERR/1

== system cddDcmApp/DataServices_DID_D934_DID_D934_2_Actual_Position/DataServices_DID_D934_DID_D934_2_Actual_Position_ReadData_sys ==
  function : TriggerPort  TriggerType=function-call  IsSimulinkFunction=on  FunctionName=DataServices_DID_D934_DID_D934_2_Actual_Position_ReadData  VariantControl=(inherit)
  Ground_1 : Ground
  Ground_2 : Ground
  Data : ArgOut  OutDataTypeStr=uint8  ArgumentName=Data
  ERR : ArgOut  Port=2  OutDataTypeStr=Std_ReturnType  ArgumentName=ERR
  wires:
  Ground_1/1 -> Data/1
  Ground_2/1 -> ERR/1

== system cddDcmApp/DataServices_DID_D935_DID_D935_1_step_Range/DataServices_DID_D935_DID_D935_1_step_Range_ReadData_sys ==
  function : TriggerPort  TriggerType=function-call  IsSimulinkFunction=on  FunctionName=DataServices_DID_D935_DID_D935_1_step_Range_ReadData  VariantControl=(inherit)
  Ground_1 : Ground
  Ground_2 : Ground
  Terminator_1 : Terminator
  OpStatus : ArgIn  OutDataTypeStr=Enum: Dcm_OpStatusType  ArgumentName=OpStatus
  Data : ArgOut  OutDataTypeStr=uint8  ArgumentName=Data
  ERR : ArgOut  Port=2  OutDataTypeStr=Std_ReturnType  ArgumentName=ERR
  wires:
  OpStatus/1 -> Terminator_1/1
  Ground_1/1 -> Data/1
  Ground_2/1 -> ERR/1

== system cddDcmApp/DataServices_DID_D935_DID_D935_1_step_Range/DataServices_DID_D935_DID_D935_1_step_Range_WriteData_sys ==
  function : TriggerPort  TriggerType=function-call  IsSimulinkFunction=on  FunctionName=DataServices_DID_D935_DID_D935_1_step_Range_WriteData  VariantControl=(inherit)
  Ground_1 : Ground
  Ground_2 : Ground
  Terminator_1 : Terminator
  Terminator_2 : Terminator
  Data : ArgIn  OutDataTypeStr=uint8  PortDimensions=2  ArgumentName=Data
  OpStatus : ArgIn  Port=2  OutDataTypeStr=Enum: Dcm_OpStatusType  ArgumentName=OpStatus
  ErrorCode : ArgOut  OutDataTypeStr=Enum: Dcm_NegativeResponseCodeType  ArgumentName=ErrorCode
  ERR : ArgOut  Port=2  OutDataTypeStr=Std_ReturnType  ArgumentName=ERR
  wires:
  Data/1 -> Terminator_1/1
  OpStatus/1 -> Terminator_2/1
  Ground_1/1 -> ErrorCode/1
  Ground_2/1 -> ERR/1

== system cddDcmApp/DataServices_DID_D935_DID_D935_2_No_Of_Movements/DataServices_DID_D935_DID_D935_2_No_Of_Movements_ReadData_sys ==
  function : TriggerPort  TriggerType=function-call  IsSimulinkFunction=on  FunctionName=DataServices_DID_D935_DID_D935_2_No_Of_Movements_ReadData  VariantControl=(inherit)
  Ground_1 : Ground
  Ground_2 : Ground
  Terminator_1 : Terminator
  OpStatus : ArgIn  OutDataTypeStr=Enum: Dcm_OpStatusType  ArgumentName=OpStatus
  Data : ArgOut  OutDataTypeStr=uint8  ArgumentName=Data
  ERR : ArgOut  Port=2  OutDataTypeStr=Std_ReturnType  ArgumentName=ERR
  wires:
  OpStatus/1 -> Terminator_1/1
  Ground_1/1 -> Data/1
  Ground_2/1 -> ERR/1

== system cddDcmApp/DataServices_DID_D935_DID_D935_2_No_Of_Movements/DataServices_DID_D935_DID_D935_2_No_Of_Movements_WriteData_sys ==
  function : TriggerPort  TriggerType=function-call  IsSimulinkFunction=on  FunctionName=DataServices_DID_D935_DID_D935_2_No_Of_Movements_WriteData  VariantControl=(inherit)
  Ground_1 : Ground
  Ground_2 : Ground
  Terminator_1 : Terminator
  Terminator_2 : Terminator
  Data : ArgIn  OutDataTypeStr=uint8  PortDimensions=4  ArgumentName=Data
  OpStatus : ArgIn  Port=2  OutDataTypeStr=Enum: Dcm_OpStatusType  ArgumentName=OpStatus
  ErrorCode : ArgOut  OutDataTypeStr=Enum: Dcm_NegativeResponseCodeType  ArgumentName=ErrorCode
  ERR : ArgOut  Port=2  OutDataTypeStr=Std_ReturnType  ArgumentName=ERR
  wires:
  Data/1 -> Terminator_1/1
  OpStatus/1 -> Terminator_2/1
  Ground_1/1 -> ErrorCode/1
  Ground_2/1 -> ERR/1

== system cddDcmApp/DataServices_DID_D936_ComplexStructure_2/DataServices_DID_D936_ComplexStructure_2_ReadData_sys ==
  function : TriggerPort  TriggerType=function-call  IsSimulinkFunction=on  FunctionName=DataServices_DID_D936_ComplexStructure_2_ReadData  VariantControl=(inherit)
  Ground_1 : Ground
  Ground_2 : Ground
  Terminator_1 : Terminator
  OpStatus : ArgIn  OutDataTypeStr=Enum: Dcm_OpStatusType  ArgumentName=OpStatus
  Data : ArgOut  OutDataTypeStr=uint8  ArgumentName=Data
  ERR : ArgOut  Port=2  OutDataTypeStr=Std_ReturnType  ArgumentName=ERR
  wires:
  OpStatus/1 -> Terminator_1/1
  Ground_1/1 -> Data/1
  Ground_2/1 -> ERR/1

== system cddDcmApp/DataServices_DID_D936_DID_D936_1_Requested_Position/DataServices_DID_D936_DID_D936_1_Requested_Position_ReadData_sys ==
  function : TriggerPort  TriggerType=function-call  IsSimulinkFunction=on  FunctionName=DataServices_DID_D936_DID_D936_1_Requested_Position_ReadData  VariantControl=(inherit)
  Ground_1 : Ground
  Ground_2 : Ground
  Terminator_1 : Terminator
  OpStatus : ArgIn  OutDataTypeStr=Enum: Dcm_OpStatusType  ArgumentName=OpStatus
  Data : ArgOut  OutDataTypeStr=uint8  ArgumentName=Data
  ERR : ArgOut  Port=2  OutDataTypeStr=Std_ReturnType  ArgumentName=ERR
  wires:
  OpStatus/1 -> Terminator_1/1
  Ground_1/1 -> Data/1
  Ground_2/1 -> ERR/1

== system cddDcmApp/DataServices_DID_D936_DID_D936_2_Actual_Position/DataServices_DID_D936_DID_D936_2_Actual_Position_ReadData_sys ==
  function : TriggerPort  TriggerType=function-call  IsSimulinkFunction=on  FunctionName=DataServices_DID_D936_DID_D936_2_Actual_Position_ReadData  VariantControl=(inherit)
  Ground_1 : Ground
  Ground_2 : Ground
  Terminator_1 : Terminator
  OpStatus : ArgIn  OutDataTypeStr=Enum: Dcm_OpStatusType  ArgumentName=OpStatus
  Data : ArgOut  OutDataTypeStr=uint8  ArgumentName=Data
  ERR : ArgOut  Port=2  OutDataTypeStr=Std_ReturnType  ArgumentName=ERR
  wires:
  OpStatus/1 -> Terminator_1/1
  Ground_1/1 -> Data/1
  Ground_2/1 -> ERR/1

== system cddDcmApp/DataServices_DID_D937_DID_D937_1_step_Range/DataServices_DID_D937_DID_D937_1_step_Range_ReadData_sys ==
  function : TriggerPort  TriggerType=function-call  IsSimulinkFunction=on  FunctionName=DataServices_DID_D937_DID_D937_1_step_Range_ReadData  VariantControl=(inherit)
  Ground_1 : Ground
  Ground_2 : Ground
  Terminator_1 : Terminator
  OpStatus : ArgIn  OutDataTypeStr=Enum: Dcm_OpStatusType  ArgumentName=OpStatus
  Data : ArgOut  OutDataTypeStr=uint8  ArgumentName=Data
  ERR : ArgOut  Port=2  OutDataTypeStr=Std_ReturnType  ArgumentName=ERR
  wires:
  OpStatus/1 -> Terminator_1/1
  Ground_1/1 -> Data/1
  Ground_2/1 -> ERR/1

== system cddDcmApp/DataServices_DID_D937_DID_D937_1_step_Range/DataServices_DID_D937_DID_D937_1_step_Range_WriteData_sys ==
  function : TriggerPort  TriggerType=function-call  IsSimulinkFunction=on  FunctionName=DataServices_DID_D937_DID_D937_1_step_Range_WriteData  VariantControl=(inherit)
  Ground_1 : Ground
  Ground_2 : Ground
  Terminator_1 : Terminator
  Terminator_2 : Terminator
  Data : ArgIn  OutDataTypeStr=uint8  PortDimensions=2  ArgumentName=Data
  OpStatus : ArgIn  Port=2  OutDataTypeStr=Enum: Dcm_OpStatusType  ArgumentName=OpStatus
  ErrorCode : ArgOut  OutDataTypeStr=Enum: Dcm_NegativeResponseCodeType  ArgumentName=ErrorCode
  ERR : ArgOut  Port=2  OutDataTypeStr=Std_ReturnType  ArgumentName=ERR
  wires:
  Data/1 -> Terminator_1/1
  OpStatus/1 -> Terminator_2/1
  Ground_1/1 -> ErrorCode/1
  Ground_2/1 -> ERR/1

== system cddDcmApp/DataServices_DID_D937_DID_D937_2_No_Of_Movements/DataServices_DID_D937_DID_D937_2_No_Of_Movements_ReadData_sys ==
  function : TriggerPort  TriggerType=function-call  IsSimulinkFunction=on  FunctionName=DataServices_DID_D937_DID_D937_2_No_Of_Movements_ReadData  VariantControl=(inherit)
  Ground_1 : Ground
  Ground_2 : Ground
  Terminator_1 : Terminator
  OpStatus : ArgIn  OutDataTypeStr=Enum: Dcm_OpStatusType  ArgumentName=OpStatus
  Data : ArgOut  OutDataTypeStr=uint8  ArgumentName=Data
  ERR : ArgOut  Port=2  OutDataTypeStr=Std_ReturnType  ArgumentName=ERR
  wires:
  OpStatus/1 -> Terminator_1/1
  Ground_1/1 -> Data/1
  Ground_2/1 -> ERR/1

== system cddDcmApp/DataServices_DID_D937_DID_D937_2_No_Of_Movements/DataServices_DID_D937_DID_D937_2_No_Of_Movements_WriteData_sys ==
  function : TriggerPort  TriggerType=function-call  IsSimulinkFunction=on  FunctionName=DataServices_DID_D937_DID_D937_2_No_Of_Movements_WriteData  VariantControl=(inherit)
  Ground_1 : Ground
  Ground_2 : Ground
  Terminator_1 : Terminator
  Terminator_2 : Terminator
  Data : ArgIn  OutDataTypeStr=uint8  PortDimensions=4  ArgumentName=Data
  OpStatus : ArgIn  Port=2  OutDataTypeStr=Enum: Dcm_OpStatusType  ArgumentName=OpStatus
  ErrorCode : ArgOut  OutDataTypeStr=Enum: Dcm_NegativeResponseCodeType  ArgumentName=ErrorCode
  ERR : ArgOut  Port=2  OutDataTypeStr=Std_ReturnType  ArgumentName=ERR
  wires:
  Data/1 -> Terminator_1/1
  OpStatus/1 -> Terminator_2/1
  Ground_1/1 -> ErrorCode/1
  Ground_2/1 -> ERR/1

== system cddDcmApp/DataServices_DID_D938_ComplexStructure_2/DataServices_DID_D938_ComplexStructure_2_ReadData_sys ==
  function : TriggerPort  TriggerType=function-call  IsSimulinkFunction=on  FunctionName=DataServices_DID_D938_ComplexStructure_2_ReadData  VariantControl=(inherit)
  Ground_1 : Ground
  Ground_2 : Ground
  Terminator_1 : Terminator
  OpStatus : ArgIn  OutDataTypeStr=Enum: Dcm_OpStatusType  ArgumentName=OpStatus
  Data : ArgOut  OutDataTypeStr=uint8  ArgumentName=Data
  ERR : ArgOut  Port=2  OutDataTypeStr=Std_ReturnType  ArgumentName=ERR
  wires:
  OpStatus/1 -> Terminator_1/1
  Ground_1/1 -> Data/1
  Ground_2/1 -> ERR/1

== system cddDcmApp/DataServices_DID_D938_DID_D938_1_Requested_Position/DataServices_DID_D938_DID_D938_1_Requested_Position_ReadData_sys ==
  function : TriggerPort  TriggerType=function-call  IsSimulinkFunction=on  FunctionName=DataServices_DID_D938_DID_D938_1_Requested_Position_ReadData  VariantControl=(inherit)
  Ground_1 : Ground
  Ground_2 : Ground
  Terminator_1 : Terminator
  OpStatus : ArgIn  OutDataTypeStr=Enum: Dcm_OpStatusType  ArgumentName=OpStatus
  Data : ArgOut  OutDataTypeStr=uint8  ArgumentName=Data
  ERR : ArgOut  Port=2  OutDataTypeStr=Std_ReturnType  ArgumentName=ERR
  wires:
  OpStatus/1 -> Terminator_1/1
  Ground_1/1 -> Data/1
  Ground_2/1 -> ERR/1

== system cddDcmApp/DataServices_DID_D938_DID_D938_2_Actual_Position/DataServices_DID_D938_DID_D938_2_Actual_Position_ReadData_sys ==
  function : TriggerPort  TriggerType=function-call  IsSimulinkFunction=on  FunctionName=DataServices_DID_D938_DID_D938_2_Actual_Position_ReadData  VariantControl=(inherit)
  Ground_1 : Ground
  Ground_2 : Ground
  Terminator_1 : Terminator
  OpStatus : ArgIn  OutDataTypeStr=Enum: Dcm_OpStatusType  ArgumentName=OpStatus
  Data : ArgOut  OutDataTypeStr=uint8  ArgumentName=Data
  ERR : ArgOut  Port=2  OutDataTypeStr=Std_ReturnType  ArgumentName=ERR
  wires:
  OpStatus/1 -> Terminator_1/1
  Ground_1/1 -> Data/1
  Ground_2/1 -> ERR/1

== system cddDcmApp/DataServices_DID_D939_DID_D939_1_step_Range/DataServices_DID_D939_DID_D939_1_step_Range_ReadData_sys ==
  function : TriggerPort  TriggerType=function-call  IsSimulinkFunction=on  FunctionName=DataServices_DID_D939_DID_D939_1_step_Range_ReadData  VariantControl=(inherit)
  Ground_1 : Ground
  Ground_2 : Ground
  Terminator_1 : Terminator
  OpStatus : ArgIn  OutDataTypeStr=Enum: Dcm_OpStatusType  ArgumentName=OpStatus
  Data : ArgOut  OutDataTypeStr=uint8  ArgumentName=Data
  ERR : ArgOut  Port=2  OutDataTypeStr=Std_ReturnType  ArgumentName=ERR
  wires:
  OpStatus/1 -> Terminator_1/1
  Ground_1/1 -> Data/1
  Ground_2/1 -> ERR/1

== system cddDcmApp/DataServices_DID_D939_DID_D939_1_step_Range/DataServices_DID_D939_DID_D939_1_step_Range_WriteData_sys ==
  function : TriggerPort  TriggerType=function-call  IsSimulinkFunction=on  FunctionName=DataServices_DID_D939_DID_D939_1_step_Range_WriteData  VariantControl=(inherit)
  Ground_1 : Ground
  Ground_2 : Ground
  Terminator_1 : Terminator
  Terminator_2 : Terminator
  Data : ArgIn  OutDataTypeStr=uint8  PortDimensions=2  ArgumentName=Data
  OpStatus : ArgIn  Port=2  OutDataTypeStr=Enum: Dcm_OpStatusType  ArgumentName=OpStatus
  ErrorCode : ArgOut  OutDataTypeStr=Enum: Dcm_NegativeResponseCodeType  ArgumentName=ErrorCode
  ERR : ArgOut  Port=2  OutDataTypeStr=Std_ReturnType  ArgumentName=ERR
  wires:
  Data/1 -> Terminator_1/1
  OpStatus/1 -> Terminator_2/1
  Ground_1/1 -> ErrorCode/1
  Ground_2/1 -> ERR/1

== system cddDcmApp/DataServices_DID_D939_DID_D939_2_No_Of_Movements/DataServices_DID_D939_DID_D939_2_No_Of_Movements_ReadData_sys ==
  function : TriggerPort  TriggerType=function-call  IsSimulinkFunction=on  FunctionName=DataServices_DID_D939_DID_D939_2_No_Of_Movements_ReadData  VariantControl=(inherit)
  Ground_1 : Ground
  Ground_2 : Ground
  Terminator_1 : Terminator
  OpStatus : ArgIn  OutDataTypeStr=Enum: Dcm_OpStatusType  ArgumentName=OpStatus
  Data : ArgOut  OutDataTypeStr=uint8  ArgumentName=Data
  ERR : ArgOut  Port=2  OutDataTypeStr=Std_ReturnType  ArgumentName=ERR
  wires:
  OpStatus/1 -> Terminator_1/1
  Ground_1/1 -> Data/1
  Ground_2/1 -> ERR/1

== system cddDcmApp/DataServices_DID_D939_DID_D939_2_No_Of_Movements/DataServices_DID_D939_DID_D939_2_No_Of_Movements_WriteData_sys ==
  function : TriggerPort  TriggerType=function-call  IsSimulinkFunction=on  FunctionName=DataServices_DID_D939_DID_D939_2_No_Of_Movements_WriteData  VariantControl=(inherit)
  Ground_1 : Ground
  Ground_2 : Ground
  Terminator_1 : Terminator
  Terminator_2 : Terminator
  Data : ArgIn  OutDataTypeStr=uint8  PortDimensions=4  ArgumentName=Data
  OpStatus : ArgIn  Port=2  OutDataTypeStr=Enum: Dcm_OpStatusType  ArgumentName=OpStatus
  ErrorCode : ArgOut  OutDataTypeStr=Enum: Dcm_NegativeResponseCodeType  ArgumentName=ErrorCode
  ERR : ArgOut  Port=2  OutDataTypeStr=Std_ReturnType  ArgumentName=ERR
  wires:
  Data/1 -> Terminator_1/1
  OpStatus/1 -> Terminator_2/1
  Ground_1/1 -> ErrorCode/1
  Ground_2/1 -> ERR/1

== system cddDcmApp/DataServices_DID_D93A_ComplexStructure_2/DataServices_DID_D93A_ComplexStructure_2_ReadData_sys ==
  function : TriggerPort  TriggerType=function-call  IsSimulinkFunction=on  FunctionName=DataServices_DID_D93A_ComplexStructure_2_ReadData  VariantControl=(inherit)
  Ground_1 : Ground
  Ground_2 : Ground
  Terminator_1 : Terminator
  OpStatus : ArgIn  OutDataTypeStr=Enum: Dcm_OpStatusType  ArgumentName=OpStatus
  Data : ArgOut  OutDataTypeStr=uint8  ArgumentName=Data
  ERR : ArgOut  Port=2  OutDataTypeStr=Std_ReturnType  ArgumentName=ERR
  wires:
  OpStatus/1 -> Terminator_1/1
  Ground_1/1 -> Data/1
  Ground_2/1 -> ERR/1

== system cddDcmApp/DataServices_DID_D93A_DID_D93A_1_Requested_Position/DataServices_DID_D93A_DID_D93A_1_Requested_Position_ReadData_sys ==
  function : TriggerPort  TriggerType=function-call  IsSimulinkFunction=on  FunctionName=DataServices_DID_D93A_DID_D93A_1_Requested_Position_ReadData  VariantControl=(inherit)
  Ground_1 : Ground
  Ground_2 : Ground
  Terminator_1 : Terminator
  OpStatus : ArgIn  OutDataTypeStr=Enum: Dcm_OpStatusType  ArgumentName=OpStatus
  Data : ArgOut  OutDataTypeStr=uint8  ArgumentName=Data
  ERR : ArgOut  Port=2  OutDataTypeStr=Std_ReturnType  ArgumentName=ERR
  wires:
  OpStatus/1 -> Terminator_1/1
  Ground_1/1 -> Data/1
  Ground_2/1 -> ERR/1
